simulink model "hyd_hyb_2_int_cont_1_5cyc"  (Simulink 8.2) (continued)
== system hyd_hyb_2_int_cont_1_5cyc/controller1/optimized controller/SOC upper 0.5/SOC upper 0.6/SOC upper 0.7/SOC under 0.8/net_col_c_d_7_8/Layer 2/LW{2,1} == (continued)
  "IW{2,1}(33,:)'" : Constant  Value=[0.26257203917708549;0.21431118789447812;-0.45138277554485901;-0.13325323548178664;-0.047189729414278986;-0.03244752289419451;-0.16801120741303177;-0.051108681937020235;0.30020793989533623;0.33792039624619141;-0.30506930948212263;0.27300091603548154;0.11456783427520295;0.2025272148063324;0.20120159555634951;-0.34626030364122201;-0.33416499791984983;0.21428425236643847;0.15141650675074611;0.3967769872059857;-0.33136184458523255;-0.31708844915183759;-0.37715726577482811;-0.29120116265154439;-0.062166430106200106;-0.20984947296030557;-0.36946881269026166;-0.089688166250396203;-0.30330015716744929;0.46576974135589871;-0.11141560233394859;-0.23377525334014285;-0.30437410720213726;-0.20362878868197468;0.22019457543474438]
      mask Display: e = 0.06;c1 = [120 150 230]/255;c2 = ([120 150 230]+0)/383;c3 = ([120 150 230]+128)/383;patch([0 1 1 0],[0 0 1 1],c1);patch([0 1 1 1-e 1-e 0+e],[0 0 1 1-e 0+e 0+e],c2);patch([0 0 1 1-e 0+e 0+e],[0 1 1 1-e 1-e 0+e],c3);plot([0 1 1 0 0],[0 0 1 1 0]);disp('weights');
  "IW{2,1}(34,:)'" : Constant  Value=[0.19971252467640216;-0.086582993102635314;-0.045986213112963102;-0.17496514808268199;0.21642410199818815;0.30122268379399197;-0.42292160067490159;0.27205623187436817;-0.38160491079019337;-0.31922772828810325;0.27370157495976671;0.349719477488458;0.084713817679184816;-0.23566225586441933;-0.078852575000660091;-0.39851087721183492;0.33223153719088222;0.10765295384301854;-0.10206405033990786;-0.065301569235525603;-0.26336732516581879;0.26398765734423063;0.26173065144312951;-0.15405207735464302;0.22691772249386599;-0.42835814744646838;0.086090585035037928;0.25029339061791894;-0.43238138706187712;0.26478813923165773;-0.23589171133766315;-0.36409163759000157;0.41906767047215154;-0.14652956713551593;0.016160467562733524]
      mask Display: e = 0.06;c1 = [120 150 230]/255;c2 = ([120 150 230]+0)/383;c3 = ([120 150 230]+128)/383;patch([0 1 1 0],[0 0 1 1],c1);patch([0 1 1 1-e 1-e 0+e],[0 0 1 1-e 0+e 0+e],c2);patch([0 0 1 1-e 0+e 0+e],[0 1 1 1-e 1-e 0+e],c3);plot([0 1 1 0 0],[0 0 1 1 0]);disp('weights');
  "IW{2,1}(35,:)'" : Constant  Value=[0.10773459757334033;0.21313649591129927;0.340334650738635;-0.33759035147143646;-0.16794460229207037;0.2563222352329369;-0.36208922744111072;-0.35243803733899859;-0.38864422529988019;-0.29348734652909164;-0.37860119099321621;-0.3078963129926145;0.11983337582567917;0.094042808254359983;-0.16033496126623314;0.27549930903254444;0.38258062353221484;-0.28296398600529937;-0.3541567031173552;0.046180682528157019;0.13202211094236579;-0.27182225384784325;-0.37957532401379518;0.20497224643781112;-0.1980359618573084;0.24377883408388004;0.40340352351589998;0.19519554896633623;-0.0051646174770529421;-0.1663241168621796;0.029272978407264345;-0.27566692133317855;0.17560454234279135;0.3818792219462257;0.078886505138233143]
      mask Display: e = 0.06;c1 = [120 150 230]/255;c2 = ([120 150 230]+0)/383;c3 = ([120 150 230]+128)/383;patch([0 1 1 0],[0 0 1 1],c1);patch([0 1 1 1-e 1-e 0+e],[0 0 1 1-e 0+e 0+e],c2);patch([0 0 1 1-e 0+e 0+e],[0 1 1 1-e 1-e 0+e],c3);plot([0 1 1 0 0],[0 0 1 1 0]);disp('weights');
  "IW{2,1}(36,:)'" : Constant  Value=[-0.069421841048907626;0.27646001990436803;-0.016464081266643751;0.015532627318338186;-0.13461948359479611;0.54825525101716832;0.044439752614915617;0.029164523396638206;-0.47916532682657265;-0.34695499774926464;-0.16426959997100143;0.25668332575168112;-0.28267673299897023;-0.18092865543030104;0.42112986624036636;0.24786057920670374;-0.31146643146013947;-0.03241339176216998;0.42284292171894183;-0.35559367082551047;0.18590636426510412;-0.49730705449220686;-0.39583758920037881;-0.25094003639352958;-0.49057013065831212;0.25765466911877727;-0.15353970084933108;0.045936149986446233;0.16655800237328125;0.14539403640304044;0.33046921495946702;0.14167805946029155;0.18090769089135039;0.018059415414365503;-0.061112553797460813]
      mask Display: e = 0.06;c1 = [120 150 230]/255;c2 = ([120 150 230]+0)/383;c3 = ([120 150 230]+128)/383;patch([0 1 1 0],[0 0 1 1],c1);patch([0 1 1 1-e 1-e 0+e],[0 0 1 1-e 0+e 0+e],c2);patch([0 0 1 1-e 0+e 0+e],[0 1 1 1-e 1-e 0+e],c3);plot([0 1 1 0 0],[0 0 1 1 0]);disp('weights');
  "IW{2,1}(37,:)'" : Constant  Value=[-0.40234680931034689;0.1286987082428438;0.21231131690383354;0.010290444448207413;-0.30168516807234813;-0.11661789784891213;0.20313156414320582;0.16521995441219595;-0.40189282201521653;0.071088852749689943;-0.1180677578828099;0.29081604807877276;-0.12898198402521688;-0.30943851852371362;-0.31911073265402667;0.41891305805213352;0.38499668053414832;-0.021384010208443549;-0.19472728064544875;-0.039028622321096759;0.057925463428703756;0.35689793245577683;0.1667136152036667;-0.24256308157537007;-0.087283419494156855;0.084704980778941841;-0.31439563384280073;0.28107320276820025;-0.042796384255496075;0.32020921197446139;-0.34490289585539852;0.31055427295305321;-0.31486492571216979;0.43893388685947371;0.43784728185845773]
      mask Display: e = 0.06;c1 = [120 150 230]/255;c2 = ([120 150 230]+0)/383;c3 = ([120 150 230]+128)/383;patch([0 1 1 0],[0 0 1 1],c1);patch([0 1 1 1-e 1-e 0+e],[0 0 1 1-e 0+e 0+e],c2);patch([0 0 1 1-e 0+e 0+e],[0 1 1 1-e 1-e 0+e],c3);plot([0 1 1 0 0],[0 0 1 1 0]);disp('weights');
  "IW{2,1}(38,:)'" : Constant  Value=[-0.40802641350542052;0.37407437363009427;0.10109492012114546;0.25798186669665563;0.13435452313380969;0.43388912598520307;-0.47258824106374414;-0.38134320277906208;0.3046498974755929;-0.11643915819166621;0.21032848519264119;0.014295097975403168;0.11628254288295209;0.32891834503887923;0.26641277021773296;-0.37174293122598834;0.110824803249835;0.49853856299440752;0.05189678312497463;0.10550957288243271;-0.052287173526751399;0.18752620430852243;-0.41191879862059272;-0.002480366033164789;-0.029825904212084153;-0.25376813647565855;-0.36599366612042505;-0.080359003929321784;-0.23945928491096516;-0.25382836358487909;-0.036218756742941845;-0.3136588875281659;-0.1691834974581988;0.35319640740577823;0.25879049126458459]
      mask Display: e = 0.06;c1 = [120 150 230]/255;c2 = ([120 150 230]+0)/383;c3 = ([120 150 230]+128)/383;patch([0 1 1 0],[0 0 1 1],c1);patch([0 1 1 1-e 1-e 0+e],[0 0 1 1-e 0+e 0+e],c2);patch([0 0 1 1-e 0+e 0+e],[0 1 1 1-e 1-e 0+e],c3);plot([0 1 1 0 0],[0 0 1 1 0]);disp('weights');
  "IW{2,1}(39,:)'" : Constant  Value=[-0.23291349991325191;0.33879926770069785;-0.030282578809925881;-0.059309990750447572;0.36658168692400978;0.21225923375314404;-0.052881594154691541;0.42337836987071581;0.040275009387894391;-0.2136807689196335;0.0069010669219072762;0.4070030916695781;-0.29469899865438193;0.16694392711594916;0.2064297904301812;-0.40663483190521599;0.46012751861911116;-0.045786296003970439;0.027142894689647496;-0.087062639710789341;0.23963814641295744;-0.030980604492919461;0.23321085390134708;0.49766807021799087;-0.2203245882473634;0.10073684851202802;-0.34932742052168997;-0.27535604946254333;-0.2414213744074287;0.43392719222310655;0.2234231062553283;0.0041057439464964015;-0.4639350136182398;0.013652728752850021;-0.37712447467779936]
      mask Display: e = 0.06;c1 = [120 150 230]/255;c2 = ([120 150 230]+0)/383;c3 = ([120 150 230]+128)/383;patch([0 1 1 0],[0 0 1 1],c1);patch([0 1 1 1-e 1-e 0+e],[0 0 1 1-e 0+e 0+e],c2);patch([0 0 1 1-e 0+e 0+e],[0 1 1 1-e 1-e 0+e],c3);plot([0 1 1 0 0],[0 0 1 1 0]);disp('weights');
  "IW{2,1}(4,:)'" : Constant  Value=[-0.17137460174058045;0.48452619427448723;0.0031776445453447085;0.40135280200544787;0.22064876120501478;0.13251184545505812;0.080334858328859643;-0.44522674048704208;-0.28422309501119603;0.24798341525772336;0.17415784202285711;0.12040076703622883;0.076516079806274118;0.41044718655903911;-0.19696390733045457;-0.07830072048616224;0.24748901612474242;0.20377376363904159;-0.13005457797874995;0.2225309475918674;-0.21236147284968512;-0.45734876239653222;0.29459638600184218;-0.45024544761886343;-0.19208139366456356;-0.44786748233473406;-0.29614198949863912;-0.11127397494107578;0.19185098726687011;0.24341594752133797;0.28443934869662474;0.10717553132779939;0.024168692578478632;0.26235031899542988;0.043681900895526878]
      mask Display: e = 0.06;c1 = [120 150 230]/255;c2 = ([120 150 230]+0)/383;c3 = ([120 150 230]+128)/383;patch([0 1 1 0],[0 0 1 1],c1);patch([0 1 1 1-e 1-e 0+e],[0 0 1 1-e 0+e 0+e],c2);patch([0 0 1 1-e 0+e 0+e],[0 1 1 1-e 1-e 0+e],c3);plot([0 1 1 0 0],[0 0 1 1 0]);disp('weights');
  "IW{2,1}(40,:)'" : Constant  Value=[0.33511713614696753;0.044764745417024292;-0.2383757079365966;0.16349577260344234;-0.14820486813114295;-0.28548986494523793;0.15125076671157839;0.096749166445875431;-0.21319290655500472;-0.25152068137149408;-0.34173049734535771;0.40981573955266876;-0.11421939653555749;-0.090037613536519248;0.36471799023468426;-0.37594796775639971;0.35260298699096104;-0.14986817058983551;-0.0053615547980489066;0.046550522623569152;-0.16548072539219141;0.32322610332080909;-0.3630232313822781;0.35234836069111936;-0.44522212432468999;0.15804535389222876;0.30495987061573698;0.36366889210948844;-0.35544882401138761;0.19558431320231148;0.1136682569164319;-0.052784590271664197;-0.14472752965333541;0.02801512535971765;-0.39277397295535033]
      mask Display: e = 0.06;c1 = [120 150 230]/255;c2 = ([120 150 230]+0)/383;c3 = ([120 150 230]+128)/383;patch([0 1 1 0],[0 0 1 1],c1);patch([0 1 1 1-e 1-e 0+e],[0 0 1 1-e 0+e 0+e],c2);patch([0 0 1 1-e 0+e 0+e],[0 1 1 1-e 1-e 0+e],c3);plot([0 1 1 0 0],[0 0 1 1 0]);disp('weights');
  "IW{2,1}(41,:)'" : Constant  Value=[-0.16697642591780953;0.16425375654796007;-0.25516508488448275;-0.37215406155092706;-0.41205506737242104;-0.20180285125891678;0.26915140547110439;-0.30928537781685894;-0.15435555661012401;0.080673258785652541;0.42688364335891532;0.43299813260125358;-0.38089365533189445;-0.33944571616046593;0.20677120714805136;-0.22240993940736306;0.22984774926901858;0.26228868438760433;0.17056288904401065;-0.2920172535504727;0.033947979919363332;-0.22871589007916235;0.078207430180461376;-0.43795284845680765;-0.096475177641642956;0.038795358310254172;0.13125249157553223;-0.34844607780717912;0.16800568490944404;-0.22266274872335973;-0.41325203277434308;0.36894622022032036;0.0064711746481903708;0.13840319092655576;0.1406505936220972]
      mask Display: e = 0.06;c1 = [120 150 230]/255;c2 = ([120 150 230]+0)/383;c3 = ([120 150 230]+128)/383;patch([0 1 1 0],[0 0 1 1],c1);patch([0 1 1 1-e 1-e 0+e],[0 0 1 1-e 0+e 0+e],c2);patch([0 0 1 1-e 0+e 0+e],[0 1 1 1-e 1-e 0+e],c3);plot([0 1 1 0 0],[0 0 1 1 0]);disp('weights');
  "IW{2,1}(42,:)'" : Constant  Value=[-0.063652203493459056;-0.35741313719530121;0.22010029871212086;0.36042866205833018;0.33120367813899165;-0.15089115794097338;0.21356271923885273;-0.31546031519329837;-0.0025202146988535547;-0.3514817302640636;0.34663348479865491;0.39811909259207617;0.062990972925501473;-0.12876603470861911;-0.1079948382606162;-0.19729046526765759;0.25998505480742473;0.13184058292773415;0.19119911896146488;0.041699644996971812;0.43413592886856273;0.39062530512344784;0.29736505548321601;-0.30392886000991959;0.17426186917825268;0.43532023653418522;-0.2740325810005525;0.21381761215319806;-0.41231185325836062;0.36420953359538377;0.17096998722102;-0.11132288661054385;0.43128089858455643;-0.051347210454415454;0.052645964552918791]
      mask Display: e = 0.06;c1 = [120 150 230]/255;c2 = ([120 150 230]+0)/383;c3 = ([120 150 230]+128)/383;patch([0 1 1 0],[0 0 1 1],c1);patch([0 1 1 1-e 1-e 0+e],[0 0 1 1-e 0+e 0+e],c2);patch([0 0 1 1-e 0+e 0+e],[0 1 1 1-e 1-e 0+e],c3);plot([0 1 1 0 0],[0 0 1 1 0]);disp('weights');
  "IW{2,1}(43,:)'" : Constant  Value=[-0.0081774130735528319;-0.30078376687700453;-0.39212715947135479;0.27237135969178056;-0.31198517438855938;-0.21467878646757349;0.1469665859318216;-0.38748333932061729;-0.094206634295571051;0.18078894611049237;0.2169628383262793;-0.36240405021888211;0.25437349994417507;0.0064580730706109988;-0.10487244273514967;-0.13451127264281493;0.28621132960999995;-0.31797789166364576;0.27792204932554454;-0.27818377902984132;-0.10949779957420536;0.25360569302584474;-0.16248339259423458;0.053068144259199458;-0.29077600236631418;-0.42243924905070046;-0.28610652352817439;-0.38498388963576696;0.3204334134027319;-0.34073710506596228;-0.26763205760915232;0.34755070994518961;-0.35428542122698276;-0.12167529469456181;0.072701931365908912]
      mask Display: e = 0.06;c1 = [120 150 230]/255;c2 = ([120 150 230]+0)/383;c3 = ([120 150 230]+128)/383;patch([0 1 1 0],[0 0 1 1],c1);patch([0 1 1 1-e 1-e 0+e],[0 0 1 1-e 0+e 0+e],c2);patch([0 0 1 1-e 0+e 0+e],[0 1 1 1-e 1-e 0+e],c3);plot([0 1 1 0 0],[0 0 1 1 0]);disp('weights');
  "IW{2,1}(44,:)'" : Constant  Value=[0.18382670824853367;-0.45131587013894719;0.22248058330606127;0.16313646770808996;0.053216581355218243;-0.1739240579509708;-0.074660903872481296;0.19406951365753314;0.34408200387046289;0.38670048898189174;0.21618526144057007;0.2521240749946993;-0.39712113792877529;0.4307608170484219;0.42985878962295287;0.37667440795493046;0.27453561813344196;0.22137079484241209;0.0057643781263494358;0.12638501201902255;-0.25693082052712363;-0.077049798629898011;0.25839465174026216;-0.11170605291453121;0.29550999497600161;0.207558728340036;-0.35042278865513643;0.014275595027265947;0.38218791597078211;0.36118814942412653;-0.057969687383922885;0.18837304823291942;0.29907005817269228;0.12482704543983195;-0.33903521264934955]
      mask Display: e = 0.06;c1 = [120 150 230]/255;c2 = ([120 150 230]+0)/383;c3 = ([120 150 230]+128)/383;patch([0 1 1 0],[0 0 1 1],c1);patch([0 1 1 1-e 1-e 0+e],[0 0 1 1-e 0+e 0+e],c2);patch([0 0 1 1-e 0+e 0+e],[0 1 1 1-e 1-e 0+e],c3);plot([0 1 1 0 0],[0 0 1 1 0]);disp('weights');
  "IW{2,1}(45,:)'" : Constant  Value=[-0.28927925305056845;0.33763201124649062;-0.25523829339035375;0.088724714777177166;0.064067092506258375;0.10147787042939832;0.11294797408465912;0.27330779459487475;-0.37410996943220209;-0.21757445508825149;-0.50978701724199826;-0.40400014230667108;-0.15192627082538848;0.1733177655604462;-0.35622242917877728;0.26124417812811529;-0.17406613013948707;-0.3561450100988568;0.11642669101693828;0.25449952694131167;-0.12283132740942704;-0.15747553572844425;0.46922888824507303;0.22336139399145233;-0.19639109478019484;-0.02485570012076312;0.22167273068331353;0.24079541198214838;-0.16724006839248989;0.14158181295980352;-0.31962437670925165;-0.30316982041205565;0.44060022230635498;0.25262737979748956;0.37541101648051117]
      mask Display: e = 0.06;c1 = [120 150 230]/255;c2 = ([120 150 230]+0)/383;c3 = ([120 150 230]+128)/383;patch([0 1 1 0],[0 0 1 1],c1);patch([0 1 1 1-e 1-e 0+e],[0 0 1 1-e 0+e 0+e],c2);patch([0 0 1 1-e 0+e 0+e],[0 1 1 1-e 1-e 0+e],c3);plot([0 1 1 0 0],[0 0 1 1 0]);disp('weights');
  "IW{2,1}(46,:)'" : Constant  Value=[0.2135980701339189;0.22943078666604541;-0.033921568539842503;0.22265613288950778;-0.12124192582469806;-0.0063680065124915915;0.032571026991028741;-0.273603275093033;0.38148559780563152;-0.12561505458676145;-0.28546858815633686;0.063674002196051016;0.18710179484864062;0.46584685287100963;-0.22830885680468443;-0.050068920815739736;0.43441193497872477;-0.31926216916277722;-0.14671829447682638;-0.29087759928359314;-0.055438419959619969;-0.17923476686673107;-0.39062297545418251;0.47190365318877731;-0.090299369859132478;-0.25407318895514847;-0.37942186832918445;0.24199611267079646;-0.0015449396286757113;-0.16580543392140146;0.20347214722436277;-0.44159864121243969;-0.33839159269183255;-0.36077025011757158;-0.39298164947706704]
      mask Display: e = 0.06;c1 = [120 150 230]/255;c2 = ([120 150 230]+0)/383;c3 = ([120 150 230]+128)/383;patch([0 1 1 0],[0 0 1 1],c1);patch([0 1 1 1-e 1-e 0+e],[0 0 1 1-e 0+e 0+e],c2);patch([0 0 1 1-e 0+e 0+e],[0 1 1 1-e 1-e 0+e],c3);plot([0 1 1 0 0],[0 0 1 1 0]);disp('weights');
  "IW{2,1}(47,:)'" : Constant  Value=[0.12108758364252444;-0.17983408020332811;-0.089163420877648267;-0.35024314161224496;0.37159707265780428;-0.14347978332741673;0.33257205273431517;-0.31760932257984609;-0.12306054554499633;0.23759904216005437;0.36095570685903788;-0.10058320572956511;-0.33894793473558882;0.037466851026924528;0.20765934709703601;-0.30691948585499274;0.30301640036482269;0.0051592138719581968;-0.27761946136723231;-0.33436713465472956;-0.33688773341933614;0.39627062316210993;-0.24956322430622785;0.20718438224187252;0.019503931277404275;-0.23785138949460213;-0.32033368207427021;0.29853141007527417;0.38609348789448933;0.23253008081360049;0.31135102413557753;0.14674560461904745;-0.3870545931080937;0.15516726089222257;0.17188996076219132]
      mask Display: e = 0.06;c1 = [120 150 230]/255;c2 = ([120 150 230]+0)/383;c3 = ([120 150 230]+128)/383;patch([0 1 1 0],[0 0 1 1],c1);patch([0 1 1 1-e 1-e 0+e],[0 0 1 1-e 0+e 0+e],c2);patch([0 0 1 1-e 0+e 0+e],[0 1 1 1-e 1-e 0+e],c3);plot([0 1 1 0 0],[0 0 1 1 0]);disp('weights');
  "IW{2,1}(48,:)'" : Constant  Value=[0.36513670380180557;0.012838082567595718;-0.35516917446542851;-0.23701731614474245;-0.12264912444737369;0.44823049681378302;-0.24620464550388571;0.1885128136444954;0.39574697779026052;-0.30091437202547061;0.35623399301049258;-0.22233628561961069;0.10223697893452358;0.20322003722789977;0.004361881257472478;0.074549710384122364;0.32089599009968045;-0.39227071484634018;0.34294003385702676;0.23351936064109374;0.07455174905963044;-0.44410141843215778;-0.26283790286921543;-0.055846211981196721;-0.1884367235873711;0.24316566588686389;-0.45222307445018528;-0.0036362311694973309;-0.068127704263345565;0.19595586414618851;0.11827176551687796;-0.21450942644750023;-0.020286281151660875;-0.34795978191273036;0.34315508917246929]
      mask Display: e = 0.06;c1 = [120 150 230]/255;c2 = ([120 150 230]+0)/383;c3 = ([120 150 230]+128)/383;patch([0 1 1 0],[0 0 1 1],c1);patch([0 1 1 1-e 1-e 0+e],[0 0 1 1-e 0+e 0+e],c2);patch([0 0 1 1-e 0+e 0+e],[0 1 1 1-e 1-e 0+e],c3);plot([0 1 1 0 0],[0 0 1 1 0]);disp('weights');
  "IW{2,1}(49,:)'" : Constant  Value=[-0.067886609658594804;-0.30192580916955714;0.28946589452121801;-0.37896137492527876;0.084702332898707322;0.31313886642379651;-0.36774066962043528;0.37802365979050467;-0.31313746480741211;-0.35376793300443349;-0.11266120528530123;-0.10524150511304403;-0.057294354426103061;-0.301219136143618;0.093820866493298835;0.32300389186525269;-0.2767434395867967;0.040354932960330665;0.17415455441887745;0.25755998228050492;0.38669737560486078;0.36646755047700341;0.26838162267020099;0.23927978518458598;0.19291641962870004;0.25811046903518364;-0.056306680582506333;0.42799506520903852;-0.37347059091709078;-0.20542769806015534;-0.45056317514593902;0.20228853076711839;-0.14816045911848399;0.16831874894077978;-0.18559029632359111]
      mask Display: e = 0.06;c1 = [120 150 230]/255;c2 = ([120 150 230]+0)/383;c3 = ([120 150 230]+128)/383;patch([0 1 1 0],[0 0 1 1],c1);patch([0 1 1 1-e 1-e 0+e],[0 0 1 1-e 0+e 0+e],c2);patch([0 0 1 1-e 0+e 0+e],[0 1 1 1-e 1-e 0+e],c3);plot([0 1 1 0 0],[0 0 1 1 0]);disp('weights');
  "IW{2,1}(5,:)'" : Constant  Value=[0.1231284690935002;-0.25667666565976288;-0.37712267664395843;0.35940673747391316;0.42763613707427117;0.26405504744145802;0.17756314879248589;0.34556207690900376;0.071390088551350067;0.033874675687618865;0.093567262408959212;0.11319645561849184;0.08135765736560438;0.13775010556099937;0.20406868835469874;0.38106610399217522;-0.039461215180228965;0.31528013113633258;0.15448531192010048;0.44682331720422852;0.26368864789829632;0.40463130911891326;-0.40959836875658862;0.22517989319414033;-0.20698591231435945;-0.26901482995153991;-0.35554574116228915;-0.3737396004541827;0.014297052187188054;0.23265303126451659;0.037171897151532796;0.30619745740339327;0.079808288907998834;0.31065962995570512;0.15455371377069121]
      mask Display: e = 0.06;c1 = [120 150 230]/255;c2 = ([120 150 230]+0)/383;c3 = ([120 150 230]+128)/383;patch([0 1 1 0],[0 0 1 1],c1);patch([0 1 1 1-e 1-e 0+e],[0 0 1 1-e 0+e 0+e],c2);patch([0 0 1 1-e 0+e 0+e],[0 1 1 1-e 1-e 0+e],c3);plot([0 1 1 0 0],[0 0 1 1 0]);disp('weights');
  "IW{2,1}(50,:)'" : Constant  Value=[0.0223731649338582;0.38840541168123627;0.27407092033259317;-0.23024431422610347;-0.25741876440440414;-0.29311093719034048;0.27071081487362025;0.31417916224786308;0.097620068336501262;0.43612693604496505;0.10268456509160478;-0.19673361633912823;-0.15659260428583871;-0.2151826211777034;0.07114785326246259;0.3114405001904319;-0.035162675803629591;-0.42405126795640502;-0.16622422735187461;0.46580635040918811;-0.0062707607248431371;-0.32335957794820674;0.11436721866510452;0.28646693635009846;0.16511039250829243;0.37198057347644703;0.20289169522147166;-0.34006719659949458;-0.26874634710527434;-0.051483253612944549;-0.32024081957992401;0.41766952195732193;0.059357495489304662;0.083941052865683791;0.39487417284890941]
      mask Display: e = 0.06;c1 = [120 150 230]/255;c2 = ([120 150 230]+0)/383;c3 = ([120 150 230]+128)/383;patch([0 1 1 0],[0 0 1 1],c1);patch([0 1 1 1-e 1-e 0+e],[0 0 1 1-e 0+e 0+e],c2);patch([0 0 1 1-e 0+e 0+e],[0 1 1 1-e 1-e 0+e],c3);plot([0 1 1 0 0],[0 0 1 1 0]);disp('weights');
  "IW{2,1}(6,:)'" : Constant  Value=[0.28730742374326002;0.13320688520531013;-0.42810368384351555;0.2126213406505098;-0.17429514508204846;-0.091065391613643423;-0.071732895526684465;-0.27231488850090002;-0.31968404262148237;-0.02772160630409784;0.1199233441353937;-0.50926665908449986;-0.18962835919821719;-0.14780958183450738;0.38014058453113014;0.11068669989526461;-0.034751909046402157;0.41052858510417861;0.16094761294159135;0.50191367516935637;0.05567212357367346;0.032882102650085301;-0.21460893392384264;-0.42336292979272072;0.079548200652746814;0.059153752471794122;0.37709423435734596;-0.29778359686107614;-0.02584513259584624;0.43843639237591542;-0.11828797876305822;0.38131434172134043;0.40510487534356698;-0.15461915278025637;0.060742817515796486]
      mask Display: e = 0.06;c1 = [120 150 230]/255;c2 = ([120 150 230]+0)/383;c3 = ([120 150 230]+128)/383;patch([0 1 1 0],[0 0 1 1],c1);patch([0 1 1 1-e 1-e 0+e],[0 0 1 1-e 0+e 0+e],c2);patch([0 0 1 1-e 0+e 0+e],[0 1 1 1-e 1-e 0+e],c3);plot([0 1 1 0 0],[0 0 1 1 0]);disp('weights');
  "IW{2,1}(7,:)'" : Constant  Value=[0.036956529768382561;-0.39972294222017629;-0.35712683257821154;0.16107407739046409;-0.017241047338916505;-0.39724176687549839;-0.31195935759527615;0.23652160771612285;0.2348502381207507;0.30669918621111936;-0.24075498329134959;-0.15033853508917547;0.26608604968035304;-0.0061298904040325824;0.10809626464211702;-0.32450703282096777;-0.32385436227834546;0.25988073038287823;0.20010431368409026;-0.22245063364146606;0.024800296995357726;-0.1296754542915316;0.26898407743090624;0.35021153713138631;-0.31768732302768488;0.28131140512788033;0.047727251204848387;-0.024278063309497545;-0.40063514868950589;-0.21392830255527345;0.27080399994584059;0.23878190583993106;-0.091654478102216219;-0.46596434452521163;-0.34728479517089478]
      mask Display: e = 0.06;c1 = [120 150 230]/255;c2 = ([120 150 230]+0)/383;c3 = ([120 150 230]+128)/383;patch([0 1 1 0],[0 0 1 1],c1);patch([0 1 1 1-e 1-e 0+e],[0 0 1 1-e 0+e 0+e],c2);patch([0 0 1 1-e 0+e 0+e],[0 1 1 1-e 1-e 0+e],c3);plot([0 1 1 0 0],[0 0 1 1 0]);disp('weights');
  "IW{2,1}(8,:)'" : Constant  Value=[0.31724906906454758;-0.40098045993299325;0.014618556219574509;0.17736550237819013;-0.063153745670256514;-0.17997710008385184;0.14675453859521431;0.11945213895342729;0.18872764232591385;0.3502555502917446;0.082261093670948277;-0.34590632588196846;-0.15239604076624524;-0.11139735451715875;-0.0043095198752764975;0.094466002851747596;-0.15029667628063073;-0.40374193030502076;0.21964912629539504;0.069896822731698088;0.44751267706305564;-0.043643600904185391;-0.086127335083810611;-0.40770522453505914;0.35546551117356145;-0.036028212126721401;0.34127963797119332;-0.44305535215558756;-0.095872019249798407;-0.4938192696913194;-0.11909515891295147;0.2979941790400995;0.4722213816045876;-0.43535867308930731;0.15442040333556947]
      mask Display: e = 0.06;c1 = [120 150 230]/255;c2 = ([120 150 230]+0)/383;c3 = ([120 150 230]+128)/383;patch([0 1 1 0],[0 0 1 1],c1);patch([0 1 1 1-e 1-e 0+e],[0 0 1 1-e 0+e 0+e],c2);patch([0 0 1 1-e 0+e 0+e],[0 1 1 1-e 1-e 0+e],c3);plot([0 1 1 0 0],[0 0 1 1 0]);disp('weights');
  "IW{2,1}(9,:)'" : Constant  Value=[-0.12787649803568557;-0.3296099537383777;-0.30490784928802173;0.29811840941112605;-0.24002979649619186;-0.22830572821886858;-0.32871429395966351;0.32577468517095132;0.31750072881603181;0.36376602825202037;0.37970674589372255;0.18101509432628166;0.2222609874619399;-0.06264579437303594;-0.12291910080710609;-0.080362913640659242;-0.32533841599570396;0.26812902795056642;0.27363941517513424;-0.38848174960740167;-0.26473608101928048;-0.36575439770835555;-0.11176180870167214;-0.32233124227502613;-0.17024784021420364;-0.25862969646977146;0.27644776241680663;0.21886318129005808;-0.13238075206720276;-0.36565056430894927;0.17054135810471102;-0.32685124058462328;0.13320789374769978;0.28714920990937703;0.081708545169553767]
      mask Display: e = 0.06;c1 = [120 150 230]/255;c2 = ([120 150 230]+0)/383;c3 = ([120 150 230]+128)/383;patch([0 1 1 0],[0 0 1 1],c1);patch([0 1 1 1-e 1-e 0+e],[0 0 1 1-e 0+e 0+e],c2);patch([0 0 1 1-e 0+e 0+e],[0 1 1 1-e 1-e 0+e],c3);plot([0 1 1 0 0],[0 0 1 1 0]);disp('weights');
  Mux : Mux  Inputs=50
      mask Display: e = 0.06;c1 = [120 150 230]/255;c2 = ([120 150 230]+0)/383;c3 = ([120 150 230]+128)/383;patch([0 1 1 0],[0 0 1 1],c1);patch([0 1 1 1-e 1-e 0+e],[0 0 1 1-e 0+e 0+e],c2);patch([0 0 1 1-e 0+e 0+e],[0 1 1 1-e 1-e 0+e],c3);plot([0 1 1 0 0],[0 0 1 1 0]);disp('Mux');
  dotprod{1..50} (50 alike) : neural/Weight Functions/dotprod  SystemSampleTime=-1
  "lz{2,1}" : Outport
      mask Display: e = 0.06;c1 = [120 230 180]/255;c2 = ([120 230 180]+0)/(256+128);c3 = ([120 230 180]+128)/(256+128);patch([0 1 1 0],[0 0 1 1],c1);patch([0 1 1 1-e 1-e 0+e],[0 0 1 1-e 0+e 0+e],c2);patch([0 0 1 1-e 0+e 0+e],[0 1 1 1-e 1-e 0+e],c3);plot([0 1 1 0 0],[0 0 1 1 0]);
  wires:
  Mux/1 -> "lz{2,1}"/1
  dotprod50/1 -> Mux/50
  "IW{2,1}(50,:)'"/1 -> dotprod50/1
  dotprod49/1 -> Mux/49
  "IW{2,1}(49,:)'"/1 -> dotprod49/1
  dotprod48/1 -> Mux/48
  "IW{2,1}(48,:)'"/1 -> dotprod48/1
  dotprod47/1 -> Mux/47
  "IW{2,1}(47,:)'"/1 -> dotprod47/1
  dotprod46/1 -> Mux/46
  "IW{2,1}(46,:)'"/1 -> dotprod46/1
  dotprod45/1 -> Mux/45
  "IW{2,1}(45,:)'"/1 -> dotprod45/1
  dotprod44/1 -> Mux/44
  "IW{2,1}(44,:)'"/1 -> dotprod44/1
  dotprod43/1 -> Mux/43
  "IW{2,1}(43,:)'"/1 -> dotprod43/1
  dotprod42/1 -> Mux/42
  "IW{2,1}(42,:)'"/1 -> dotprod42/1
  dotprod41/1 -> Mux/41
  "IW{2,1}(41,:)'"/1 -> dotprod41/1
  dotprod40/1 -> Mux/40
  "IW{2,1}(40,:)'"/1 -> dotprod40/1
  dotprod39/1 -> Mux/39
  "IW{2,1}(39,:)'"/1 -> dotprod39/1
  dotprod38/1 -> Mux/38
  "IW{2,1}(38,:)'"/1 -> dotprod38/1
  dotprod37/1 -> Mux/37
  "IW{2,1}(37,:)'"/1 -> dotprod37/1
  dotprod36/1 -> Mux/36
  "IW{2,1}(36,:)'"/1 -> dotprod36/1
  dotprod35/1 -> Mux/35
  "IW{2,1}(35,:)'"/1 -> dotprod35/1
  dotprod34/1 -> Mux/34
  "IW{2,1}(34,:)'"/1 -> dotprod34/1
  dotprod33/1 -> Mux/33
  "IW{2,1}(33,:)'"/1 -> dotprod33/1
  dotprod32/1 -> Mux/32
  "IW{2,1}(32,:)'"/1 -> dotprod32/1
  dotprod31/1 -> Mux/31
  "IW{2,1}(31,:)'"/1 -> dotprod31/1
  dotprod30/1 -> Mux/30
  "IW{2,1}(30,:)'"/1 -> dotprod30/1
  dotprod29/1 -> Mux/29
  "IW{2,1}(29,:)'"/1 -> dotprod29/1
  dotprod28/1 -> Mux/28
  "IW{2,1}(28,:)'"/1 -> dotprod28/1
  dotprod27/1 -> Mux/27
  "IW{2,1}(27,:)'"/1 -> dotprod27/1
  dotprod26/1 -> Mux/26
  "IW{2,1}(26,:)'"/1 -> dotprod26/1
  dotprod25/1 -> Mux/25
  "IW{2,1}(25,:)'"/1 -> dotprod25/1
  dotprod24/1 -> Mux/24
  "IW{2,1}(24,:)'"/1 -> dotprod24/1
  dotprod23/1 -> Mux/23
  "IW{2,1}(23,:)'"/1 -> dotprod23/1
  dotprod22/1 -> Mux/22
  "IW{2,1}(22,:)'"/1 -> dotprod22/1
  dotprod21/1 -> Mux/21
  "IW{2,1}(21,:)'"/1 -> dotprod21/1
  dotprod20/1 -> Mux/20
  "IW{2,1}(20,:)'"/1 -> dotprod20/1
  dotprod19/1 -> Mux/19
  "IW{2,1}(19,:)'"/1 -> dotprod19/1
  dotprod18/1 -> Mux/18
  "IW{2,1}(18,:)'"/1 -> dotprod18/1
  dotprod17/1 -> Mux/17
  "IW{2,1}(17,:)'"/1 -> dotprod17/1
  dotprod16/1 -> Mux/16
  "IW{2,1}(16,:)'"/1 -> dotprod16/1
  dotprod15/1 -> Mux/15
  "IW{2,1}(15,:)'"/1 -> dotprod15/1
  dotprod14/1 -> Mux/14
  "IW{2,1}(14,:)'"/1 -> dotprod14/1
  dotprod13/1 -> Mux/13
  "IW{2,1}(13,:)'"/1 -> dotprod13/1
  dotprod12/1 -> Mux/12
  "IW{2,1}(12,:)'"/1 -> dotprod12/1
  dotprod11/1 -> Mux/11
  "IW{2,1}(11,:)'"/1 -> dotprod11/1
  dotprod10/1 -> Mux/10
  "IW{2,1}(10,:)'"/1 -> dotprod10/1
  dotprod9/1 -> Mux/9
  "IW{2,1}(9,:)'"/1 -> dotprod9/1
  dotprod8/1 -> Mux/8
  "IW{2,1}(8,:)'"/1 -> dotprod8/1
  dotprod7/1 -> Mux/7
  "IW{2,1}(7,:)'"/1 -> dotprod7/1
  dotprod6/1 -> Mux/6
  "IW{2,1}(6,:)'"/1 -> dotprod6/1
  dotprod5/1 -> Mux/5
  "IW{2,1}(5,:)'"/1 -> dotprod5/1
  dotprod4/1 -> Mux/4
  "IW{2,1}(4,:)'"/1 -> dotprod4/1
  dotprod3/1 -> Mux/3
  "IW{2,1}(3,:)'"/1 -> dotprod3/1
  dotprod2/1 -> Mux/2
  "IW{2,1}(2,:)'"/1 -> dotprod2/1
  dotprod1/1 -> Mux/1
  "ad{2,1}"/1 -> dotprod50/2, dotprod49/2, dotprod48/2, dotprod47/2, dotprod46/2, dotprod45/2, dotprod44/2, dotprod43/2, dotprod42/2, dotprod41/2, dotprod40/2, dotprod39/2, dotprod38/2, dotprod37/2, dotprod36/2, dotprod35/2, dotprod34/2, dotprod33/2, dotprod32/2, dotprod31/2, dotprod30/2, dotprod29/2, dotprod28/2, dotprod27/2, dotprod26/2, dotprod25/2, dotprod24/2, dotprod23/2, dotprod22/2, dotprod21/2, dotprod20/2, dotprod19/2, dotprod18/2, dotprod17/2, dotprod16/2, dotprod15/2, dotprod14/2, dotprod13/2, dotprod12/2, dotprod11/2, dotprod10/2, dotprod9/2, dotprod8/2, dotprod7/2, dotprod6/2, dotprod5/2, dotprod4/2, dotprod3/2, dotprod2/2, dotprod1/2
  "IW{2,1}(1,:)'"/1 -> dotprod1/1
